simulink model "Demo_PreScanCarRealTime2D_cs"  (Simulink 8.0) (continued)
== system Demo_PreScanCarRealTime2D_cs/HostVehicle/Dynamics_UserSpecified/VI-CarRealTime/Output_Bus/IOmap2bus == (continued)
  Demux/471 -> Steering_Instant_Axis_Rotation/2  "R1"
  System/1 -> OutputBus/50  "System"
  Demux/472 -> System/1  "Angular_Acceleration_Norm"
  Demux/473 -> System/2  "Angular_Speed_Norm"
  Demux/474 -> System/3  "Translational_Acceleration_Norm"
  Demux/475 -> System/4  "Translational_Speed_Norm"
  System_Mass_Center/1 -> OutputBus/51  "System_Mass_Center"
  Demux/476 -> System_Mass_Center/1  "Global_Ax"
  Demux/477 -> System_Mass_Center/2  "Global_Ay"
  Demux/478 -> System_Mass_Center/3  "Global_Az"
  Demux/479 -> System_Mass_Center/4  "Global_Vx"
  Demux/480 -> System_Mass_Center/5  "Global_Vy"
  Demux/481 -> System_Mass_Center/6  "Global_Vz"
  Demux/482 -> System_Mass_Center/7  "Global_X"
  Demux/483 -> System_Mass_Center/8  "Global_Y"
  Demux/484 -> System_Mass_Center/9  "Global_Z"
  Demux/485 -> System_Mass_Center/10  "Normal_Acceleration"
  Demux/486 -> System_Mass_Center/11  "Path_Curvature"
  Demux/487 -> System_Mass_Center/12  "Speed"
  Demux/488 -> System_Mass_Center/13  "Tangential_Acceleration"
  til_wheel_tire_forces/1 -> OutputBus/52  "til_wheel_tire_forces"
  Demux/489 -> til_wheel_tire_forces/1  "aligning_torque_front"
  Demux/490 -> til_wheel_tire_forces/2  "aligning_torque_rear"
  Demux/491 -> til_wheel_tire_forces/3  "aligning_torque_twin_rear"
  Demux/492 -> til_wheel_tire_forces/4  "lateral_front"
  Demux/493 -> til_wheel_tire_forces/5  "lateral_rear"
  Demux/494 -> til_wheel_tire_forces/6  "lateral_twin_rear"
  Demux/495 -> til_wheel_tire_forces/7  "longitudinal_front"
  Demux/496 -> til_wheel_tire_forces/8  "longitudinal_rear"
  Demux/497 -> til_wheel_tire_forces/9  "longitudinal_twin_rear"
  Demux/498 -> til_wheel_tire_forces/10  "normal_front"
  Demux/499 -> til_wheel_tire_forces/11  "normal_rear"
  Demux/500 -> til_wheel_tire_forces/12  "normal_twin_rear"
  Demux/501 -> til_wheel_tire_forces/13  "overturning_moment_front"
  Demux/502 -> til_wheel_tire_forces/14  "overturning_moment_rear"
  Demux/503 -> til_wheel_tire_forces/15  "overturning_moment_twin_rear"
  Demux/504 -> til_wheel_tire_forces/16  "rolling_resistance_front"
  Demux/505 -> til_wheel_tire_forces/17  "rolling_resistance_rear"
  Demux/506 -> til_wheel_tire_forces/18  "rolling_resistance_twin_rear"
  til_wheel_tire_kinematics/1 -> OutputBus/53  "til_wheel_tire_kinematics"
  Demux/507 -> til_wheel_tire_kinematics/1  "inclination_angle_front"
  Demux/508 -> til_wheel_tire_kinematics/2  "inclination_angle_rear"
  Demux/509 -> til_wheel_tire_kinematics/3  "inclination_angle_twin_rear"
  Demux/510 -> til_wheel_tire_kinematics/4  "lateral_slip_front"
  Demux/511 -> til_wheel_tire_kinematics/5  "lateral_slip_rear"
  Demux/512 -> til_wheel_tire_kinematics/6  "lateral_slip_twin_rear"
  Demux/513 -> til_wheel_tire_kinematics/7  "longitudinal_slip_front"
  Demux/514 -> til_wheel_tire_kinematics/8  "longitudinal_slip_rear"
  Demux/515 -> til_wheel_tire_kinematics/9  "longitudinal_slip_twin_rear"
  til_wheel_tire_rolling_states/1 -> OutputBus/54  "til_wheel_tire_rolling_states"
  Demux/516 -> til_wheel_tire_rolling_states/1  "omega_actual_front"
  Demux/517 -> til_wheel_tire_rolling_states/2  "omega_actual_rear"
  Demux/518 -> til_wheel_tire_rolling_states/3  "omega_actual_twin_rear"
  Demux/519 -> til_wheel_tire_rolling_states/4  "omega_free_front"
  Demux/520 -> til_wheel_tire_rolling_states/5  "omega_free_rear"
  Demux/521 -> til_wheel_tire_rolling_states/6  "omega_free_twin_rear"
  Demux/522 -> til_wheel_tire_rolling_states/7  "rolling_radius_front"
  Demux/523 -> til_wheel_tire_rolling_states/8  "rolling_radius_rear"
  Demux/524 -> til_wheel_tire_rolling_states/9  "rolling_radius_twin_rear"
  Tire/1 -> OutputBus/55  "Tire"
  Actual_Tread_Speed/1 -> Tire/1  "Actual_Tread_Speed"
  Demux/525 -> Actual_Tread_Speed/1
  Demux/526 -> Actual_Tread_Speed/2
  Demux/527 -> Actual_Tread_Speed/3  "R1"
  Demux/528 -> Actual_Tread_Speed/4  "R2"
  Contact_Patch_Global_dZdX/1 -> Tire/2  "Contact_Patch_Global_dZdX"
  Demux/529 -> Contact_Patch_Global_dZdX/1
  Demux/530 -> Contact_Patch_Global_dZdX/2
  Demux/531 -> Contact_Patch_Global_dZdX/3  "R1"
  Demux/532 -> Contact_Patch_Global_dZdX/4  "R2"
  Contact_Patch_Global_dZdY/1 -> Tire/3  "Contact_Patch_Global_dZdY"
  Demux/533 -> Contact_Patch_Global_dZdY/1
  Demux/534 -> Contact_Patch_Global_dZdY/2
  Demux/535 -> Contact_Patch_Global_dZdY/3  "R1"
  Demux/536 -> Contact_Patch_Global_dZdY/4  "R2"
  Contact_Patch_Global_X/1 -> Tire/4  "Contact_Patch_Global_X"
  Demux/537 -> Contact_Patch_Global_X/1
  Demux/538 -> Contact_Patch_Global_X/2
  Demux/539 -> Contact_Patch_Global_X/3  "R1"
  Demux/540 -> Contact_Patch_Global_X/4  "R2"
  Contact_Patch_Global_Y/1 -> Tire/5  "Contact_Patch_Global_Y"
  Demux/541 -> Contact_Patch_Global_Y/1
  Demux/542 -> Contact_Patch_Global_Y/2
  Demux/543 -> Contact_Patch_Global_Y/3  "R1"
  Demux/544 -> Contact_Patch_Global_Y/4  "R2"
  Contact_Patch_Global_Z/1 -> Tire/6  "Contact_Patch_Global_Z"
  Demux/545 -> Contact_Patch_Global_Z/1
  Demux/546 -> Contact_Patch_Global_Z/2
  Demux/547 -> Contact_Patch_Global_Z/3  "R1"
  Demux/548 -> Contact_Patch_Global_Z/4  "R2"
  Effective_Closing_Speed/1 -> Tire/7  "Effective_Closing_Speed"
  Demux/549 -> Effective_Closing_Speed/1
  Demux/550 -> Effective_Closing_Speed/2
  Demux/551 -> Effective_Closing_Speed/3  "R1"
  Demux/552 -> Effective_Closing_Speed/4  "R2"
  Effective_Penetration/1 -> Tire/8  "Effective_Penetration"
  Demux/553 -> Effective_Penetration/1
  Demux/554 -> Effective_Penetration/2
  Demux/555 -> Effective_Penetration/3  "R1"
  Demux/556 -> Effective_Penetration/4  "R2"
  Effective_Rolling_Radius/1 -> Tire/9  "Effective_Rolling_Radius"
  Demux/557 -> Effective_Rolling_Radius/1
  Demux/558 -> Effective_Rolling_Radius/2
  Demux/559 -> Effective_Rolling_Radius/3  "R1"
  Demux/560 -> Effective_Rolling_Radius/4  "R2"
  Equivalent_Tread_Speed/1 -> Tire/10  "Equivalent_Tread_Speed"
  Demux/561 -> Equivalent_Tread_Speed/1
  Demux/562 -> Equivalent_Tread_Speed/2
  Demux/563 -> Equivalent_Tread_Speed/3  "R1"
  Demux/564 -> Equivalent_Tread_Speed/4  "R2"
  External_Friction_Scale_Factor/1 -> Tire/11  "External_Friction_Scale_Factor"
  Demux/565 -> External_Friction_Scale_Factor/1
  Demux/566 -> External_Friction_Scale_Factor/2
  Demux/567 -> External_Friction_Scale_Factor/3  "R1"
  Demux/568 -> External_Friction_Scale_Factor/4  "R2"
  Ground_Surface_Contact_Patch_Vx/1 -> Tire/12  "Ground_Surface_Contact_Patch_Vx"
  Demux/569 -> Ground_Surface_Contact_Patch_Vx/1
  Demux/570 -> Ground_Surface_Contact_Patch_Vx/2
  Demux/571 -> Ground_Surface_Contact_Patch_Vx/3  "R1"
  Demux/572 -> Ground_Surface_Contact_Patch_Vx/4  "R2"
  Ground_Surface_Contact_Patch_Vy/1 -> Tire/13  "Ground_Surface_Contact_Patch_Vy"
  Demux/573 -> Ground_Surface_Contact_Patch_Vy/1
  Demux/574 -> Ground_Surface_Contact_Patch_Vy/2
  Demux/575 -> Ground_Surface_Contact_Patch_Vy/3  "R1"
  Demux/576 -> Ground_Surface_Contact_Patch_Vy/4  "R2"
  Ground_Surface_Force_X/1 -> Tire/14  "Ground_Surface_Force_X"
  Demux/577 -> Ground_Surface_Force_X/1
  Demux/578 -> Ground_Surface_Force_X/2
  Demux/579 -> Ground_Surface_Force_X/3  "R1"
  Demux/580 -> Ground_Surface_Force_X/4  "R2"
  Ground_Surface_Force_Y/1 -> Tire/15  "Ground_Surface_Force_Y"
  Demux/581 -> Ground_Surface_Force_Y/1
  Demux/582 -> Ground_Surface_Force_Y/2
  Demux/583 -> Ground_Surface_Force_Y/3  "R1"
  Demux/584 -> Ground_Surface_Force_Y/4  "R2"
  Ground_Surface_Force_Z/1 -> Tire/16  "Ground_Surface_Force_Z"
  Demux/585 -> Ground_Surface_Force_Z/1
  Demux/586 -> Ground_Surface_Force_Z/2
  Demux/587 -> Ground_Surface_Force_Z/3  "R1"
  Demux/588 -> Ground_Surface_Force_Z/4  "R2"
  Ground_Surface_Gyroscopic_Moment/1 -> Tire/17  "Ground_Surface_Gyroscopic_Moment"
  Demux/589 -> Ground_Surface_Gyroscopic_Moment/1
  Demux/590 -> Ground_Surface_Gyroscopic_Moment/2
  Demux/591 -> Ground_Surface_Gyroscopic_Moment/3  "R1"
  Demux/592 -> Ground_Surface_Gyroscopic_Moment/4  "R2"
  Ground_Surface_Kappa_Fy/1 -> Tire/18  "Ground_Surface_Kappa_Fy"
  Demux/593 -> Ground_Surface_Kappa_Fy/1
  Demux/594 -> Ground_Surface_Kappa_Fy/2
  Demux/595 -> Ground_Surface_Kappa_Fy/3  "R1"
  Demux/596 -> Ground_Surface_Kappa_Fy/4  "R2"
  Ground_Surface_Moment_X/1 -> Tire/19  "Ground_Surface_Moment_X"
  Demux/597 -> Ground_Surface_Moment_X/1
  Demux/598 -> Ground_Surface_Moment_X/2
  Demux/599 -> Ground_Surface_Moment_X/3  "R1"
  Demux/600 -> Ground_Surface_Moment_X/4  "R2"
  Ground_Surface_Moment_Y/1 -> Tire/20  "Ground_Surface_Moment_Y"
  Demux/601 -> Ground_Surface_Moment_Y/1
  Demux/602 -> Ground_Surface_Moment_Y/2
  Demux/603 -> Ground_Surface_Moment_Y/3  "R1"
  Demux/604 -> Ground_Surface_Moment_Y/4  "R2"
  Ground_Surface_Moment_Z/1 -> Tire/21  "Ground_Surface_Moment_Z"
  Demux/605 -> Ground_Surface_Moment_Z/1
  Demux/606 -> Ground_Surface_Moment_Z/2
  Demux/607 -> Ground_Surface_Moment_Z/3  "R1"
  Demux/608 -> Ground_Surface_Moment_Z/4  "R2"
  Ground_Surface_Peak_Friction_X/1 -> Tire/22  "Ground_Surface_Peak_Friction_X"
  Demux/609 -> Ground_Surface_Peak_Friction_X/1
  Demux/610 -> Ground_Surface_Peak_Friction_X/2
  Demux/611 -> Ground_Surface_Peak_Friction_X/3  "R1"
  Demux/612 -> Ground_Surface_Peak_Friction_X/4  "R2"
  Ground_Surface_Peak_Friction_Y/1 -> Tire/23  "Ground_Surface_Peak_Friction_Y"
  Demux/613 -> Ground_Surface_Peak_Friction_Y/1
  Demux/614 -> Ground_Surface_Peak_Friction_Y/2
  Demux/615 -> Ground_Surface_Peak_Friction_Y/3  "R1"
  Demux/616 -> Ground_Surface_Peak_Friction_Y/4  "R2"
  Ground_Surface_Residual_Torque/1 -> Tire/24  "Ground_Surface_Residual_Torque"
  Demux/617 -> Ground_Surface_Residual_Torque/1
  Demux/618 -> Ground_Surface_Residual_Torque/2
  Demux/619 -> Ground_Surface_Residual_Torque/3  "R1"
  Demux/620 -> Ground_Surface_Residual_Torque/4  "R2"
  Hub_Carrier_Force_Global_X/1 -> Tire/25  "Hub_Carrier_Force_Global_X"
  Demux/621 -> Hub_Carrier_Force_Global_X/1
  Demux/622 -> Hub_Carrier_Force_Global_X/2
  Demux/623 -> Hub_Carrier_Force_Global_X/3  "R1"
  Demux/624 -> Hub_Carrier_Force_Global_X/4  "R2"
  Hub_Carrier_Force_Global_Y/1 -> Tire/26  "Hub_Carrier_Force_Global_Y"
  Demux/625 -> Hub_Carrier_Force_Global_Y/1
  Demux/626 -> Hub_Carrier_Force_Global_Y/2
  Demux/627 -> Hub_Carrier_Force_Global_Y/3  "R1"
  Demux/628 -> Hub_Carrier_Force_Global_Y/4  "R2"
  Hub_Carrier_Force_Global_Z/1 -> Tire/27  "Hub_Carrier_Force_Global_Z"
  Demux/629 -> Hub_Carrier_Force_Global_Z/1
  Demux/630 -> Hub_Carrier_Force_Global_Z/2
  Demux/631 -> Hub_Carrier_Force_Global_Z/3  "R1"
  Demux/632 -> Hub_Carrier_Force_Global_Z/4  "R2"
  Hub_Carrier_Force_Local_X/1 -> Tire/28  "Hub_Carrier_Force_Local_X"
  Demux/633 -> Hub_Carrier_Force_Local_X/1
  Demux/634 -> Hub_Carrier_Force_Local_X/2
  Demux/635 -> Hub_Carrier_Force_Local_X/3  "R1"
  Demux/636 -> Hub_Carrier_Force_Local_X/4  "R2"
  Hub_Carrier_Force_Local_Y/1 -> Tire/29  "Hub_Carrier_Force_Local_Y"
  Demux/637 -> Hub_Carrier_Force_Local_Y/1
  Demux/638 -> Hub_Carrier_Force_Local_Y/2
  Demux/639 -> Hub_Carrier_Force_Local_Y/3  "R1"
  Demux/640 -> Hub_Carrier_Force_Local_Y/4  "R2"
  Hub_Carrier_Force_Local_Z/1 -> Tire/30  "Hub_Carrier_Force_Local_Z"
  Demux/641 -> Hub_Carrier_Force_Local_Z/1
  Demux/642 -> Hub_Carrier_Force_Local_Z/2
  Demux/643 -> Hub_Carrier_Force_Local_Z/3  "R1"
  Demux/644 -> Hub_Carrier_Force_Local_Z/4  "R2"
  Hub_Carrier_Moment_Global_X/1 -> Tire/31  "Hub_Carrier_Moment_Global_X"
  Demux/645 -> Hub_Carrier_Moment_Global_X/1
  Demux/646 -> Hub_Carrier_Moment_Global_X/2
  Demux/647 -> Hub_Carrier_Moment_Global_X/3  "R1"
  Demux/648 -> Hub_Carrier_Moment_Global_X/4  "R2"
  Hub_Carrier_Moment_Global_Y/1 -> Tire/32  "Hub_Carrier_Moment_Global_Y"
  Demux/649 -> Hub_Carrier_Moment_Global_Y/1
  Demux/650 -> Hub_Carrier_Moment_Global_Y/2
  Demux/651 -> Hub_Carrier_Moment_Global_Y/3  "R1"
  Demux/652 -> Hub_Carrier_Moment_Global_Y/4  "R2"
  Hub_Carrier_Moment_Global_Z/1 -> Tire/33  "Hub_Carrier_Moment_Global_Z"
  Demux/653 -> Hub_Carrier_Moment_Global_Z/1
  Demux/654 -> Hub_Carrier_Moment_Global_Z/2
  Demux/655 -> Hub_Carrier_Moment_Global_Z/3  "R1"
  Demux/656 -> Hub_Carrier_Moment_Global_Z/4  "R2"
  Hub_Carrier_Moment_Local_X/1 -> Tire/34  "Hub_Carrier_Moment_Local_X"
  Demux/657 -> Hub_Carrier_Moment_Local_X/1
  Demux/658 -> Hub_Carrier_Moment_Local_X/2
  Demux/659 -> Hub_Carrier_Moment_Local_X/3  "R1"
  Demux/660 -> Hub_Carrier_Moment_Local_X/4  "R2"
  Hub_Carrier_Moment_Local_Y/1 -> Tire/35  "Hub_Carrier_Moment_Local_Y"
  Demux/661 -> Hub_Carrier_Moment_Local_Y/1
  Demux/662 -> Hub_Carrier_Moment_Local_Y/2
  Demux/663 -> Hub_Carrier_Moment_Local_Y/3  "R1"
  Demux/664 -> Hub_Carrier_Moment_Local_Y/4  "R2"
  Hub_Carrier_Moment_Local_Z/1 -> Tire/36  "Hub_Carrier_Moment_Local_Z"
  Demux/665 -> Hub_Carrier_Moment_Local_Z/1
  Demux/666 -> Hub_Carrier_Moment_Local_Z/2
  Demux/667 -> Hub_Carrier_Moment_Local_Z/3  "R1"
  Demux/668 -> Hub_Carrier_Moment_Local_Z/4  "R2"
  Lateral_Relaxation_Length/1 -> Tire/37  "Lateral_Relaxation_Length"
  Demux/669 -> Lateral_Relaxation_Length/1
  Demux/670 -> Lateral_Relaxation_Length/2
  Demux/671 -> Lateral_Relaxation_Length/3  "R1"
  Demux/672 -> Lateral_Relaxation_Length/4  "R2"
  Lateral_Slip_Without_Lag/1 -> Tire/38  "Lateral_Slip_Without_Lag"
  Demux/673 -> Lateral_Slip_Without_Lag/1
  Demux/674 -> Lateral_Slip_Without_Lag/2
  Demux/675 -> Lateral_Slip_Without_Lag/3  "R1"
  Demux/676 -> Lateral_Slip_Without_Lag/4  "R2"
  Lateral_Slip_With_Lag/1 -> Tire/39  "Lateral_Slip_With_Lag"
  Demux/677 -> Lateral_Slip_With_Lag/1
  Demux/678 -> Lateral_Slip_With_Lag/2
  Demux/679 -> Lateral_Slip_With_Lag/3  "R1"
  Demux/680 -> Lateral_Slip_With_Lag/4  "R2"
  Loaded_Radius/1 -> Tire/40  "Loaded_Radius"
  Demux/681 -> Loaded_Radius/1
  Demux/682 -> Loaded_Radius/2
  Demux/683 -> Loaded_Radius/3  "R1"
  Demux/684 -> Loaded_Radius/4  "R2"
  Longitudinal_Relaxation_Length/1 -> Tire/41  "Longitudinal_Relaxation_Length"
  Demux/685 -> Longitudinal_Relaxation_Length/1
  Demux/686 -> Longitudinal_Relaxation_Length/2
  Demux/687 -> Longitudinal_Relaxation_Length/3  "R1"
  Demux/688 -> Longitudinal_Relaxation_Length/4  "R2"
  Longitudinal_Slip_Without_Lag/1 -> Tire/42  "Longitudinal_Slip_Without_Lag"
  Demux/689 -> Longitudinal_Slip_Without_Lag/1
  Demux/690 -> Longitudinal_Slip_Without_Lag/2
  Demux/691 -> Longitudinal_Slip_Without_Lag/3  "R1"
  Demux/692 -> Longitudinal_Slip_Without_Lag/4  "R2"
  Longitudinal_Slip_With_Lag/1 -> Tire/43  "Longitudinal_Slip_With_Lag"
  Demux/693 -> Longitudinal_Slip_With_Lag/1
  Demux/694 -> Longitudinal_Slip_With_Lag/2
  Demux/695 -> Longitudinal_Slip_With_Lag/3  "R1"
  Demux/696 -> Longitudinal_Slip_With_Lag/4  "R2"
  Moment_Arm_Of_Fx/1 -> Tire/44  "Moment_Arm_Of_Fx"
  Demux/697 -> Moment_Arm_Of_Fx/1
  Demux/698 -> Moment_Arm_Of_Fx/2
  Demux/699 -> Moment_Arm_Of_Fx/3  "R1"
  Demux/700 -> Moment_Arm_Of_Fx/4  "R2"
  Pneumatic_Trail/1 -> Tire/45  "Pneumatic_Trail"
  Demux/701 -> Pneumatic_Trail/1
  Demux/702 -> Pneumatic_Trail/2
  Demux/703 -> Pneumatic_Trail/3  "R1"
  Demux/704 -> Pneumatic_Trail/4  "R2"
  User_Input_Contact_Patch_Global_Z_Perturbation/1 -> Tire/46  "User_Input_Contact_Patch_Global_Z_Perturbation"
  Demux/705 -> User_Input_Contact_Patch_Global_Z_Perturbation/1
  Demux/706 -> User_Input_Contact_Patch_Global_Z_Perturbation/2
  Demux/707 -> User_Input_Contact_Patch_Global_Z_Perturbation/3  "R1"
  Demux/708 -> User_Input_Contact_Patch_Global_Z_Perturbation/4  "R2"
  tir_wheel_tire_forces/1 -> OutputBus/56  "tir_wheel_tire_forces"
  Demux/709 -> tir_wheel_tire_forces/1  "aligning_torque_front"
  Demux/710 -> tir_wheel_tire_forces/2  "aligning_torque_rear"
  Demux/711 -> tir_wheel_tire_forces/3  "aligning_torque_twin_rear"
  Demux/712 -> tir_wheel_tire_forces/4  "lateral_front"
  Demux/713 -> tir_wheel_tire_forces/5  "lateral_rear"
  Demux/714 -> tir_wheel_tire_forces/6  "lateral_twin_rear"
  Demux/715 -> tir_wheel_tire_forces/7  "longitudinal_front"
  Demux/716 -> tir_wheel_tire_forces/8  "longitudinal_rear"
  Demux/717 -> tir_wheel_tire_forces/9  "longitudinal_twin_rear"
  Demux/718 -> tir_wheel_tire_forces/10  "normal_front"
  Demux/719 -> tir_wheel_tire_forces/11  "normal_rear"
  Demux/720 -> tir_wheel_tire_forces/12  "normal_twin_rear"
  Demux/721 -> tir_wheel_tire_forces/13  "overturning_moment_front"
  Demux/722 -> tir_wheel_tire_forces/14  "overturning_moment_rear"
  Demux/723 -> tir_wheel_tire_forces/15  "overturning_moment_twin_rear"
  Demux/724 -> tir_wheel_tire_forces/16  "rolling_resistance_front"
  Demux/725 -> tir_wheel_tire_forces/17  "rolling_resistance_rear"
  Demux/726 -> tir_wheel_tire_forces/18  "rolling_resistance_twin_rear"
  tir_wheel_tire_kinematics/1 -> OutputBus/57  "tir_wheel_tire_kinematics"
  Demux/727 -> tir_wheel_tire_kinematics/1  "inclination_angle_front"
  Demux/728 -> tir_wheel_tire_kinematics/2  "inclination_angle_rear"
  Demux/729 -> tir_wheel_tire_kinematics/3  "inclination_angle_twin_rear"
  Demux/730 -> tir_wheel_tire_kinematics/4  "lateral_slip_front"
  Demux/731 -> tir_wheel_tire_kinematics/5  "lateral_slip_rear"
  Demux/732 -> tir_wheel_tire_kinematics/6  "lateral_slip_twin_rear"
  Demux/733 -> tir_wheel_tire_kinematics/7  "longitudinal_slip_front"
  Demux/734 -> tir_wheel_tire_kinematics/8  "longitudinal_slip_rear"
  Demux/735 -> tir_wheel_tire_kinematics/9  "longitudinal_slip_twin_rear"
  tir_wheel_tire_rolling_states/1 -> OutputBus/58  "tir_wheel_tire_rolling_states"
  Demux/736 -> tir_wheel_tire_rolling_states/1  "omega_actual_front"
  Demux/737 -> tir_wheel_tire_rolling_states/2  "omega_actual_rear"
  Demux/738 -> tir_wheel_tire_rolling_states/3  "omega_actual_twin_rear"
  Demux/739 -> tir_wheel_tire_rolling_states/4  "omega_free_front"
  Demux/740 -> tir_wheel_tire_rolling_states/5  "omega_free_rear"
  Demux/741 -> tir_wheel_tire_rolling_states/6  "omega_free_twin_rear"
  Demux/742 -> tir_wheel_tire_rolling_states/7  "rolling_radius_front"
  Demux/743 -> tir_wheel_tire_rolling_states/8  "rolling_radius_rear"
  Demux/744 -> tir_wheel_tire_rolling_states/9  "rolling_radius_twin_rear"
  transmission/1 -> OutputBus/59  "transmission"
  Demux/745 -> transmission/1  "gear"
  Demux/746 -> transmission/2  "input_rpm"
  Demux/747 -> transmission/3  "output_rpm"
  Demux/748 -> transmission/4  "ratio"
  ues_third_spring_data/1 -> OutputBus/60  "ues_third_spring_data"
  Demux/749 -> ues_third_spring_data/1  "displacement_front"
  Demux/750 -> ues_third_spring_data/2  "displacement_rear"
  Demux/751 -> ues_third_spring_data/3  "force_front"
  Demux/752 -> ues_third_spring_data/4  "force_rear"
  Demux/753 -> ues_third_spring_data/5  "velocity_front"
  Demux/754 -> ues_third_spring_data/6  "velocity_rear"
  upright_accelerometer/1 -> OutputBus/61  "upright_accelerometer"
  Demux/755 -> upright_accelerometer/1  "Z_left_front"
  Demux/756 -> upright_accelerometer/2  "Z_left_rear"
  Demux/757 -> upright_accelerometer/3  "Z_right_front"
  Demux/758 -> upright_accelerometer/4  "Z_right_rear"
  Vehicle/1 -> OutputBus/62  "Vehicle"
  Demux/759 -> Vehicle/1  "Body_Fixed_Alpha_X"
  Demux/760 -> Vehicle/2  "Body_Fixed_Alpha_Y"
  Demux/761 -> Vehicle/3  "Body_Fixed_Alpha_Z"
  Demux/762 -> Vehicle/4  "Body_Fixed_Omega_X"
  Demux/763 -> Vehicle/5  "Body_Fixed_Omega_Y"
  Demux/764 -> Vehicle/6  "Body_Fixed_Omega_Z"
  Demux/765 -> Vehicle/7  "CM_Body_Fixed_Ax"
  Demux/766 -> Vehicle/8  "CM_Body_Fixed_Ay"
  Demux/767 -> Vehicle/9  "CM_Body_Fixed_Az"
  Demux/768 -> Vehicle/10  "CM_Body_Fixed_Vx"
  Demux/769 -> Vehicle/11  "CM_Body_Fixed_Vy"
  Demux/770 -> Vehicle/12  "CM_Body_Fixed_Vz"
  Demux/771 -> Vehicle/13  "CM_Global_And_Tracking_Az"
  Demux/772 -> Vehicle/14  "CM_Global_And_Tracking_Vz"
  Demux/773 -> Vehicle/15  "CM_Global_Ax"
  Demux/774 -> Vehicle/16  "CM_Global_Ay"
  Demux/775 -> Vehicle/17  "CM_Global_Vx"
  Demux/776 -> Vehicle/18  "CM_Global_Vy"
  Demux/777 -> Vehicle/19  "CM_Global_X"
  Demux/778 -> Vehicle/20  "CM_Global_Y"
  Demux/779 -> Vehicle/21  "CM_Global_Z"
  Demux/780 -> Vehicle/22  "CM_Tracking_Ax"
  Demux/781 -> Vehicle/23  "CM_Tracking_Ay"
  Demux/782 -> Vehicle/24  "CM_Tracking_Vx"
  Demux/783 -> Vehicle/25  "CM_Tracking_Vy"
  Demux/784 -> Vehicle/26  "cross_weight_PID"
  Demux/785 -> Vehicle/27  "Euler_Roll"
  Demux/786 -> Vehicle/28  "front_ride_height_at_center_point"
  Demux/787 -> Vehicle/29  "Global_Alpha_X"
  Demux/788 -> Vehicle/30  "Global_Alpha_Y"
  Demux/789 -> Vehicle/31  "Global_Alpha_Z"
  Demux/790 -> Vehicle/32  "Global_Omega_X"
  Demux/791 -> Vehicle/33  "Global_Omega_Y"
  Demux/792 -> Vehicle/34  "Global_Omega_Z"
  Demux/793 -> Vehicle/35  "Inclination_Roll"
  Demux/794 -> Vehicle/36  "Origin_Distance_Traveled"
  Demux/795 -> Vehicle/37  "Origin_Global_X"
  Demux/796 -> Vehicle/38  "Origin_Global_Y"
  Demux/797 -> Vehicle/39  "Origin_Global_Z"
  Demux/798 -> Vehicle/40  "Origin_Global_Z_wrt_road_mk"
  Demux/799 -> Vehicle/41  "Pitch"
  Demux/800 -> Vehicle/42  "Side_Slip_Angle"
  Demux/801 -> Vehicle/43  "Side_Slip_Angle_Rate"
  Demux/802 -> Vehicle/44  "Understeer_Gradient"
  Demux/803 -> Vehicle/45  "Understeer_Stability_Factor"
  Demux/804 -> Vehicle/46  "Yaw"
  Vehicle_States/1 -> OutputBus/63  "Vehicle_States"
  Demux/805 -> Vehicle_States/1  "horizontal_vel_wrt_road"
  Demux/806 -> Vehicle_States/2  "lateral_acc_wrt_road"
  Demux/807 -> Vehicle_States/3  "lateral_disp"
  Demux/808 -> Vehicle_States/4  "lateral_vel_wrt_road"
  Demux/809 -> Vehicle_States/5  "longitudinal_acc_wrt_road"
  Demux/810 -> Vehicle_States/6  "longitudinal_disp"
  Demux/811 -> Vehicle_States/7  "longitudinal_vel_wrt_road"
  Demux/812 -> Vehicle_States/8  "pitch_angle"
  Demux/813 -> Vehicle_States/9  "pitch_angular_acc_wrt_road"
  Demux/814 -> Vehicle_States/10  "pitch_angular_vel_wrt_road"
  Demux/815 -> Vehicle_States/11  "roll_angle"
  Demux/816 -> Vehicle_States/12  "roll_angular_acc_wrt_road"
  Demux/817 -> Vehicle_States/13  "roll_angular_vel_wrt_road"
  Demux/818 -> Vehicle_States/14  "side_slip"
  Demux/819 -> Vehicle_States/15  "vertical_acc_wrt_road"
  Demux/820 -> Vehicle_States/16  "vertical_disp"
  Demux/821 -> Vehicle_States/17  "vertical_vel_wrt_road"
  Demux/822 -> Vehicle_States/18  "yaw_angle"
  Demux/823 -> Vehicle_States/19  "yaw_angular_acc_wrt_road"
  Demux/824 -> Vehicle_States/20  "yaw_angular_vel_wrt_road"
  Wheel/1 -> OutputBus/64  "Wheel"
  Alpha/1 -> Wheel/1  "Alpha"
  Demux/825 -> Alpha/1
  Demux/826 -> Alpha/2
  Demux/827 -> Alpha/3  "R1"
  Demux/828 -> Alpha/4  "R2"
  Caster_Angle/1 -> Wheel/2  "Caster_Angle"
  Demux/829 -> Caster_Angle/1  "left"
  Demux/830 -> Caster_Angle/2  "right"
  Drive_Moment/1 -> Wheel/3  "Drive_Moment"
  Demux/831 -> Drive_Moment/1
  Demux/832 -> Drive_Moment/2
  Demux/833 -> Drive_Moment/3  "R1"
  Demux/834 -> Drive_Moment/4  "R2"
  Euler_Roll/1 -> Wheel/4  "Euler_Roll"
  Demux/835 -> Euler_Roll/1
  Demux/836 -> Euler_Roll/2
  Demux/837 -> Euler_Roll/3  "R1"
  Demux/838 -> Euler_Roll/4  "R2"
  Global_Omega_X/1 -> Wheel/5  "Global_Omega_X"
  Demux/839 -> Global_Omega_X/1
  Demux/840 -> Global_Omega_X/2
  Demux/841 -> Global_Omega_X/3  "R1"
  Demux/842 -> Global_Omega_X/4  "R2"
  Global_Omega_Y/1 -> Wheel/6  "Global_Omega_Y"
  Demux/843 -> Global_Omega_Y/1
  Demux/844 -> Global_Omega_Y/2
  Demux/845 -> Global_Omega_Y/3  "R1"
  Demux/846 -> Global_Omega_Y/4  "R2"
  Global_Omega_Z/1 -> Wheel/7  "Global_Omega_Z"
  Demux/847 -> Global_Omega_Z/1
  Demux/848 -> Global_Omega_Z/2
  Demux/849 -> Global_Omega_Z/3  "R1"
  Demux/850 -> Global_Omega_Z/4  "R2"
  Global_Vx/1 -> Wheel/8  "Global_Vx"
  Demux/851 -> Global_Vx/1
  Demux/852 -> Global_Vx/2
  Demux/853 -> Global_Vx/3  "R1"
  Demux/854 -> Global_Vx/4  "R2"
  Global_Vy/1 -> Wheel/9  "Global_Vy"
  Demux/855 -> Global_Vy/1
  Demux/856 -> Global_Vy/2
  Demux/857 -> Global_Vy/3  "R1"
  Demux/858 -> Global_Vy/4  "R2"
  Global_Vz/1 -> Wheel/10  "Global_Vz"
  Demux/859 -> Global_Vz/1
  Demux/860 -> Global_Vz/2
  Demux/861 -> Global_Vz/3  "R1"
  Demux/862 -> Global_Vz/4  "R2"
  Global_X/1 -> Wheel/11  "Global_X"
  Demux/863 -> Global_X/1
  Demux/864 -> Global_X/2
  Demux/865 -> Global_X/3  "R1"
  Demux/866 -> Global_X/4  "R2"
  Global_Y/1 -> Wheel/12  "Global_Y"
  Demux/867 -> Global_Y/1
  Demux/868 -> Global_Y/2
  Demux/869 -> Global_Y/3  "R1"
  Demux/870 -> Global_Y/4  "R2"
  Global_Z/1 -> Wheel/13  "Global_Z"
  Demux/871 -> Global_Z/1
  Demux/872 -> Global_Z/2
  Demux/873 -> Global_Z/3  "R1"
  Demux/874 -> Global_Z/4  "R2"
  Ground_Surface_Wheel_Center_Vx/1 -> Wheel/14  "Ground_Surface_Wheel_Center_Vx"
  Demux/875 -> Ground_Surface_Wheel_Center_Vx/1
  Demux/876 -> Ground_Surface_Wheel_Center_Vx/2
  Demux/877 -> Ground_Surface_Wheel_Center_Vx/3  "R1"
  Demux/878 -> Ground_Surface_Wheel_Center_Vx/4  "R2"
  Hub_Carrier_Omega_X/1 -> Wheel/15  "Hub_Carrier_Omega_X"
  Demux/879 -> Hub_Carrier_Omega_X/1
  Demux/880 -> Hub_Carrier_Omega_X/2
  Demux/881 -> Hub_Carrier_Omega_X/3  "R1"
  Demux/882 -> Hub_Carrier_Omega_X/4  "R2"
  Hub_Carrier_Omega_Y/1 -> Wheel/16  "Hub_Carrier_Omega_Y"
  Demux/883 -> Hub_Carrier_Omega_Y/1
  Demux/884 -> Hub_Carrier_Omega_Y/2
  Demux/885 -> Hub_Carrier_Omega_Y/3  "R1"
  Demux/886 -> Hub_Carrier_Omega_Y/4  "R2"
  Hub_Carrier_Omega_Z/1 -> Wheel/17  "Hub_Carrier_Omega_Z"
  Demux/887 -> Hub_Carrier_Omega_Z/1
  Demux/888 -> Hub_Carrier_Omega_Z/2
  Demux/889 -> Hub_Carrier_Omega_Z/3  "R1"
  Demux/890 -> Hub_Carrier_Omega_Z/4  "R2"
  Hub_Carrier_Vx/1 -> Wheel/18  "Hub_Carrier_Vx"
  Demux/891 -> Hub_Carrier_Vx/1
  Demux/892 -> Hub_Carrier_Vx/2
  Demux/893 -> Hub_Carrier_Vx/3  "R1"
  Demux/894 -> Hub_Carrier_Vx/4  "R2"
  Hub_Carrier_Vy/1 -> Wheel/19  "Hub_Carrier_Vy"
  Demux/895 -> Hub_Carrier_Vy/1
  Demux/896 -> Hub_Carrier_Vy/2
  Demux/897 -> Hub_Carrier_Vy/3  "R1"
  Demux/898 -> Hub_Carrier_Vy/4  "R2"
  Hub_Carrier_Vz/1 -> Wheel/20  "Hub_Carrier_Vz"
  Demux/899 -> Hub_Carrier_Vz/1
  Demux/900 -> Hub_Carrier_Vz/2
  Demux/901 -> Hub_Carrier_Vz/3  "R1"
  Demux/902 -> Hub_Carrier_Vz/4  "R2"
  hub_center_accx/1 -> Wheel/21  "hub_center_accx"
  Demux/903 -> hub_center_accx/1  "front_left"
  Demux/904 -> hub_center_accx/2  "front_right"
  Demux/905 -> hub_center_accx/3  "rear_left"
  Demux/906 -> hub_center_accx/4  "rear_right"
  hub_center_accy/1 -> Wheel/22  "hub_center_accy"
  Demux/907 -> hub_center_accy/1  "front_left"
  Demux/908 -> hub_center_accy/2  "front_right"
  Demux/909 -> hub_center_accy/3  "rear_left"
  Demux/910 -> hub_center_accy/4  "rear_right"
  hub_center_accz/1 -> Wheel/23  "hub_center_accz"
  Demux/911 -> hub_center_accz/1  "front_left"
  Demux/912 -> hub_center_accz/2  "front_right"
  Demux/913 -> hub_center_accz/3  "rear_left"
  Demux/914 -> hub_center_accz/4  "rear_right"
  Inclination/1 -> Wheel/24  "Inclination"
  Demux/915 -> Inclination/1
  Demux/916 -> Inclination/2
  Demux/917 -> Inclination/3  "R1"
  Demux/918 -> Inclination/4  "R2"
  Kingpin_Moment1/1 -> Wheel/25  "Kingpin_Moment"
  Demux/919 -> Kingpin_Moment1/1
  Demux/920 -> Kingpin_Moment1/2  "R1"
  Omega/1 -> Wheel/26  "Omega"
  Demux/921 -> Omega/1
  Demux/922 -> Omega/2
  Demux/923 -> Omega/3  "R1"
  Demux/924 -> Omega/4  "R2"
  Phi/1 -> Wheel/27  "Phi"
  Demux/925 -> Phi/1
  Demux/926 -> Phi/2
  Demux/927 -> Phi/3  "R1"
  Demux/928 -> Phi/4  "R2"
  Pitch/1 -> Wheel/28  "Pitch"
  Demux/929 -> Pitch/1
  Demux/930 -> Pitch/2
  Demux/931 -> Pitch/3  "R1"
  Demux/932 -> Pitch/4  "R2"
  Psi/1 -> Wheel/29  "Psi"
  Demux/933 -> Psi/1
  Demux/934 -> Psi/2
  Demux/935 -> Psi/3  "R1"
  Demux/936 -> Psi/4  "R2"
  Rotation/1 -> Wheel/30  "Rotation"
  Demux/937 -> Rotation/1
  Demux/938 -> Rotation/2
  Demux/939 -> Rotation/3  "R1"
  Demux/940 -> Rotation/4  "R2"
  Side_View_Angle/1 -> Wheel/31  "Side_View_Angle"
  Demux/941 -> Side_View_Angle/1
  Demux/942 -> Side_View_Angle/2
  Demux/943 -> Side_View_Angle/3  "R1"
  Demux/944 -> Side_View_Angle/4  "R2"
  Spindle_Steer/1 -> Wheel/32  "Spindle_Steer"
  Demux/945 -> Spindle_Steer/1
  Demux/946 -> Spindle_Steer/2
  Demux/947 -> Spindle_Steer/3  "R1"
  Demux/948 -> Spindle_Steer/4  "R2"
  Theta/1 -> Wheel/33  "Theta"
  Demux/949 -> Theta/1
  Demux/950 -> Theta/2
  Demux/951 -> Theta/3  "R1"
  Demux/952 -> Theta/4  "R2"
  wheel_center_dispx/1 -> Wheel/34  "wheel_center_dispx"
  Demux/953 -> wheel_center_dispx/1  "front_left"
  Demux/954 -> wheel_center_dispx/2  "front_right"
  Demux/955 -> wheel_center_dispx/3  "rear_left"
  Demux/956 -> wheel_center_dispx/4  "rear_right"
  wheel_center_dispy/1 -> Wheel/35  "wheel_center_dispy"
  Demux/957 -> wheel_center_dispy/1  "front_left"
  Demux/958 -> wheel_center_dispy/2  "front_right"
  Demux/959 -> wheel_center_dispy/3  "rear_left"
  Demux/960 -> wheel_center_dispy/4  "rear_right"
  wheel_center_dispz/1 -> Wheel/36  "wheel_center_dispz"
  Demux/961 -> wheel_center_dispz/1  "front_left"
  Demux/962 -> wheel_center_dispz/2  "front_right"
  Demux/963 -> wheel_center_dispz/3  "rear_left"
  Demux/964 -> wheel_center_dispz/4  "rear_right"
  Yaw/1 -> Wheel/37  "Yaw"
  Demux/965 -> Yaw/1
  Demux/966 -> Yaw/2
  Demux/967 -> Yaw/3  "R1"
  Demux/968 -> Yaw/4  "R2"
  wheel_angles/1 -> OutputBus/65  "wheel_angles"
  Demux/969 -> wheel_angles/1  "camber_L1"
  Demux/970 -> wheel_angles/2  "camber_L2"
  Demux/971 -> wheel_angles/3  "camber_R1"
  Demux/972 -> wheel_angles/4  "camber_R2"
  Demux/973 -> wheel_angles/5  "caster_L1"
  Demux/974 -> wheel_angles/6  "caster_L2"
  Demux/975 -> wheel_angles/7  "caster_R1"
  Demux/976 -> wheel_angles/8  "caster_R2"
  Demux/977 -> wheel_angles/9  "toe_L1"
  Demux/978 -> wheel_angles/10  "toe_L2"
  Demux/979 -> wheel_angles/11  "toe_R1"
  Demux/980 -> wheel_angles/12  "toe_R2"
  whl_front_wheel_XFORM/1 -> OutputBus/66  "whl_front_wheel_XFORM"
  Demux/981 -> whl_front_wheel_XFORM/1  "FX"
  Demux/982 -> whl_front_wheel_XFORM/2  "FY"
  Demux/983 -> whl_front_wheel_XFORM/3  "FZ"
  Demux/984 -> whl_front_wheel_XFORM/4  "Phi"
  Demux/985 -> whl_front_wheel_XFORM/5  "Psi"
  Demux/986 -> whl_front_wheel_XFORM/6  "Theta"
  Demux/987 -> whl_front_wheel_XFORM/7  "X"
  Demux/988 -> whl_front_wheel_XFORM/8  "Y"
  Demux/989 -> whl_front_wheel_XFORM/9  "Z"
  whl_rear_wheel_XFORM/1 -> OutputBus/67  "whl_rear_wheel_XFORM"
  Demux/990 -> whl_rear_wheel_XFORM/1  "FX"
  Demux/991 -> whl_rear_wheel_XFORM/2  "FY"
  Demux/992 -> whl_rear_wheel_XFORM/3  "FZ"
  Demux/993 -> whl_rear_wheel_XFORM/4  "Phi"
  Demux/994 -> whl_rear_wheel_XFORM/5  "Psi"
  Demux/995 -> whl_rear_wheel_XFORM/6  "Theta"
  Demux/996 -> whl_rear_wheel_XFORM/7  "X"
  Demux/997 -> whl_rear_wheel_XFORM/8  "Y"
  Demux/998 -> whl_rear_wheel_XFORM/9  "Z"
  whl_twin_rear_wheel_XFORM/1 -> OutputBus/68  "whl_twin_rear_wheel_XFORM"
  Demux/999 -> whl_twin_rear_wheel_XFORM/1  "FX"
  Demux/1000 -> whl_twin_rear_wheel_XFORM/2  "FY"
  Demux/1001 -> whl_twin_rear_wheel_XFORM/3  "FZ"
  Demux/1002 -> whl_twin_rear_wheel_XFORM/4  "Phi"
  Demux/1003 -> whl_twin_rear_wheel_XFORM/5  "Psi"
  Demux/1004 -> whl_twin_rear_wheel_XFORM/6  "Theta"
  Demux/1005 -> whl_twin_rear_wheel_XFORM/7  "X"
  Demux/1006 -> whl_twin_rear_wheel_XFORM/8  "Y"
  Demux/1007 -> whl_twin_rear_wheel_XFORM/9  "Z"
  whr_front_wheel_XFORM/1 -> OutputBus/69  "whr_front_wheel_XFORM"
  Demux/1008 -> whr_front_wheel_XFORM/1  "FX"
  Demux/1009 -> whr_front_wheel_XFORM/2  "FY"
  Demux/1010 -> whr_front_wheel_XFORM/3  "FZ"
  Demux/1011 -> whr_front_wheel_XFORM/4  "Phi"
  Demux/1012 -> whr_front_wheel_XFORM/5  "Psi"
  Demux/1013 -> whr_front_wheel_XFORM/6  "Theta"
  Demux/1014 -> whr_front_wheel_XFORM/7  "X"
  Demux/1015 -> whr_front_wheel_XFORM/8  "Y"
  Demux/1016 -> whr_front_wheel_XFORM/9  "Z"
  whr_rear_wheel_XFORM/1 -> OutputBus/70  "whr_rear_wheel_XFORM"
  Demux/1017 -> whr_rear_wheel_XFORM/1  "FX"
  Demux/1018 -> whr_rear_wheel_XFORM/2  "FY"
  Demux/1019 -> whr_rear_wheel_XFORM/3  "FZ"
  Demux/1020 -> whr_rear_wheel_XFORM/4  "Phi"
  Demux/1021 -> whr_rear_wheel_XFORM/5  "Psi"
  Demux/1022 -> whr_rear_wheel_XFORM/6  "Theta"
  Demux/1023 -> whr_rear_wheel_XFORM/7  "X"
  Demux/1024 -> whr_rear_wheel_XFORM/8  "Y"
  Demux/1025 -> whr_rear_wheel_XFORM/9  "Z"
  whr_twin_rear_wheel_XFORM/1 -> OutputBus/71  "whr_twin_rear_wheel_XFORM"
  Demux/1026 -> whr_twin_rear_wheel_XFORM/1  "FX"
  Demux/1027 -> whr_twin_rear_wheel_XFORM/2  "FY"
  Demux/1028 -> whr_twin_rear_wheel_XFORM/3  "FZ"
  Demux/1029 -> whr_twin_rear_wheel_XFORM/4  "Phi"
  Demux/1030 -> whr_twin_rear_wheel_XFORM/5  "Psi"
  Demux/1031 -> whr_twin_rear_wheel_XFORM/6  "Theta"
  Demux/1032 -> whr_twin_rear_wheel_XFORM/7  "X"
  Demux/1033 -> whr_twin_rear_wheel_XFORM/8  "Y"
  Demux/1034 -> whr_twin_rear_wheel_XFORM/9  "Z"
  Animator_Widget/1 -> OutputBus/72  "Animator_Widget"
  Demux/1035 -> Animator_Widget/1  "longitudinal_speed"
  Demux/1036 -> Animator_Widget/2  "engine_rpm"
  ges_hub_XFORMFL/1 -> OutputBus/73  "ges_hub_XFORMFL"
  Demux/1037 -> ges_hub_XFORMFL/1  "X"
  Demux/1038 -> ges_hub_XFORMFL/2  "Y"
  Demux/1039 -> ges_hub_XFORMFL/3  "Z"
  Demux/1040 -> ges_hub_XFORMFL/4  "Psi"
  Demux/1041 -> ges_hub_XFORMFL/5  "Theta"
  Demux/1042 -> ges_hub_XFORMFL/6  "Phi"
  hub2cp_XFORMFL/1 -> OutputBus/74  "hub2cp_XFORMFL"
  Demux/1043 -> hub2cp_XFORMFL/1  "X"
  Demux/1044 -> hub2cp_XFORMFL/2  "Y"
  Demux/1045 -> hub2cp_XFORMFL/3  "Z"
  cp_force_longitudinalFL/1 -> OutputBus/75  "cp_force_longitudinalFL"
  Demux/1046 -> cp_force_longitudinalFL/1  "X"
  Demux/1047 -> cp_force_longitudinalFL/2  "Y"
  Demux/1048 -> cp_force_longitudinalFL/3  "Z"
  cp_force_lateralFL/1 -> OutputBus/76  "cp_force_lateralFL"
  Demux/1049 -> cp_force_lateralFL/1  "X"
  Demux/1050 -> cp_force_lateralFL/2  "Y"
  Demux/1051 -> cp_force_lateralFL/3  "Z"
  cp_force_normalFL/1 -> OutputBus/77  "cp_force_normalFL"
  Demux/1052 -> cp_force_normalFL/1  "X"
  Demux/1053 -> cp_force_normalFL/2  "Y"
  Demux/1054 -> cp_force_normalFL/3  "Z"
  ges_hub_XFORMFR/1 -> OutputBus/78  "ges_hub_XFORMFR"
  Demux/1055 -> ges_hub_XFORMFR/1  "X"
  Demux/1056 -> ges_hub_XFORMFR/2  "Y"
  Demux/1057 -> ges_hub_XFORMFR/3  "Z"
  Demux/1058 -> ges_hub_XFORMFR/4  "Psi"
  Demux/1059 -> ges_hub_XFORMFR/5  "Theta"
  Demux/1060 -> ges_hub_XFORMFR/6  "Phi"
  hub2cp_XFORMFR/1 -> OutputBus/79  "hub2cp_XFORMFR"
  Demux/1061 -> hub2cp_XFORMFR/1  "X"
  Demux/1062 -> hub2cp_XFORMFR/2  "Y"
  Demux/1063 -> hub2cp_XFORMFR/3  "Z"
  cp_force_longitudinalFR/1 -> OutputBus/80  "cp_force_longitudinalFR"
  Demux/1064 -> cp_force_longitudinalFR/1  "X"
  Demux/1065 -> cp_force_longitudinalFR/2  "Y"
  Demux/1066 -> cp_force_longitudinalFR/3  "Z"
  cp_force_lateralFR/1 -> OutputBus/81  "cp_force_lateralFR"
  Demux/1067 -> cp_force_lateralFR/1  "X"
  Demux/1068 -> cp_force_lateralFR/2  "Y"
  Demux/1069 -> cp_force_lateralFR/3  "Z"
  cp_force_normalFR/1 -> OutputBus/82  "cp_force_normalFR"
  Demux/1070 -> cp_force_normalFR/1  "X"
  Demux/1071 -> cp_force_normalFR/2  "Y"
  Demux/1072 -> cp_force_normalFR/3  "Z"
  ges_hub_XFORMRL/1 -> OutputBus/83  "ges_hub_XFORMRL"
  Demux/1073 -> ges_hub_XFORMRL/1  "X"
  Demux/1074 -> ges_hub_XFORMRL/2  "Y"
  Demux/1075 -> ges_hub_XFORMRL/3  "Z"
  Demux/1076 -> ges_hub_XFORMRL/4  "Psi"
  Demux/1077 -> ges_hub_XFORMRL/5  "Theta"
  Demux/1078 -> ges_hub_XFORMRL/6  "Phi"
  hub2cp_XFORMRL/1 -> OutputBus/84  "hub2cp_XFORMRL"
  Demux/1079 -> hub2cp_XFORMRL/1  "X"
  Demux/1080 -> hub2cp_XFORMRL/2  "Y"
  Demux/1081 -> hub2cp_XFORMRL/3  "Z"
  cp_force_longitudinalRL/1 -> OutputBus/85  "cp_force_longitudinalRL"
  Demux/1082 -> cp_force_longitudinalRL/1  "X"
  Demux/1083 -> cp_force_longitudinalRL/2  "Y"
  Demux/1084 -> cp_force_longitudinalRL/3  "Z"
  cp_force_lateralRL/1 -> OutputBus/86  "cp_force_lateralRL"
  Demux/1085 -> cp_force_lateralRL/1  "X"
  Demux/1086 -> cp_force_lateralRL/2  "Y"
  Demux/1087 -> cp_force_lateralRL/3  "Z"
  cp_force_normalRL/1 -> OutputBus/87  "cp_force_normalRL"
  Demux/1088 -> cp_force_normalRL/1  "X"
  Demux/1089 -> cp_force_normalRL/2  "Y"
  Demux/1090 -> cp_force_normalRL/3  "Z"
  ges_hub_XFORMRR/1 -> OutputBus/88  "ges_hub_XFORMRR"
  Demux/1091 -> ges_hub_XFORMRR/1  "X"
  Demux/1092 -> ges_hub_XFORMRR/2  "Y"
  Demux/1093 -> ges_hub_XFORMRR/3  "Z"
  Demux/1094 -> ges_hub_XFORMRR/4  "Psi"
  Demux/1095 -> ges_hub_XFORMRR/5  "Theta"
  Demux/1096 -> ges_hub_XFORMRR/6  "Phi"
  hub2cp_XFORMRR/1 -> OutputBus/89  "hub2cp_XFORMRR"
  Demux/1097 -> hub2cp_XFORMRR/1  "X"
  Demux/1098 -> hub2cp_XFORMRR/2  "Y"
  Demux/1099 -> hub2cp_XFORMRR/3  "Z"
  cp_force_longitudinalRR/1 -> OutputBus/90  "cp_force_longitudinalRR"
  Demux/1100 -> cp_force_longitudinalRR/1  "X"
  Demux/1101 -> cp_force_longitudinalRR/2  "Y"
  Demux/1102 -> cp_force_longitudinalRR/3  "Z"
  cp_force_lateralRR/1 -> OutputBus/91  "cp_force_lateralRR"
  Demux/1103 -> cp_force_lateralRR/1  "X"
  Demux/1104 -> cp_force_lateralRR/2  "Y"
  Demux/1105 -> cp_force_lateralRR/3  "Z"
  cp_force_normalRR/1 -> OutputBus/92  "cp_force_normalRR"
  Demux/1106 -> cp_force_normalRR/1  "X"
  Demux/1107 -> cp_force_normalRR/2  "Y"
  Demux/1108 -> cp_force_normalRR/3  "Z"
